simulink model "Lab2_simulink_plc"  (R2023b)
settings: Solver=FixedStepAuto  StartTime=0.0  StopTime=inf  RelTol=1e-3
InitFcn:
  | d13_init.PRE = 50;
undefined symbols (not defined in the file or in its folder): BOOL, DINT, OTL, slplc
libraries: arduinolib x9
(part 1 of 31)
settings Model: PLCLadderLogicDebug=off  PLCLadderLogicAnimation=on  PLCLadderLogicDataShow=off  ExtModeTrigDuration=2  Creator=pontus  LastModifiedBy=pontus
settings SolverCC: AllowMultiTaskInputOutput=on  ConcurrentTasks=on  AutoInsertRateTranBlk=on
settings OptimizationCC: EnhancedBackFolding=on  PassReuseOutputArgsAs=Individual arguments  ZeroExternalMemoryAtStartup=off  ZeroInternalMemoryAtStartup=off  MaxStackSize=64  OptimizeBlockOrder=speed  DifferentSizesBufferReuse=on  OptimizationCustomize=off  EfficientTunableParamExpr=on
settings DebuggingCC: ParameterTunabilityLossMsg=error  BusObjectLabelMismatch=error
settings HardwareCC: ProdBitPerInt=16  ProdBitPerPointer=16  ProdBitPerSizeT=16  ProdBitPerPtrDiffT=16  ProdLargestAtomicFloat=None  ProdWordSize=8  ProdHWDeviceType=Atmel->AVR  UseEmbeddedCoderFeatures=off  UseSimulinkCoderFeatures=off
settings SFSimCC: MATLABDynamicMemAlloc=off
settings RTWCC: Description=Embedded Coder  SystemTargetFile=ert.tlc  HardwareBoard=Arduino Uno  TemplateMakefile=ert_default_tmf  Toolchain=Arduino AVR
settings CodeAppCC: ForceParamTrailComments=on  IgnoreCustomStorageClasses=off  OperatorAnnotations=on  SimulinkDataObjDesc=on  SFDataObjDesc=on  InsertBlockDesc=on
settings ERTTargetCC: SupportContinuousTime=on  SupportNonInlinedSFcns=on  SupportVariableSizeSignals=on  ExtModeTransport=3  ExtModeStaticAlloc=on  ExtModeAutomaticAllocSize=off  ExtModeStaticAllocSize=250  ExtModeMexFile=ext_xcp  ExtModeIntrfLevel=Level2 - Open
settings ConfigComp: PLC_TargetIDE=studio5000  PLC_MultiTBSigbuilderTimeRange=off  PLC_FoldFBCallOutputVar=off  PLC_RemoveUnusedSSMethodCallArguments=off  PLC_UseCallOutputArgSyntax=off  PLC_EnableUnsignedAndDoubleTypes=off  PLC_EnableUDTSplitting=off  PLC_MaxFieldsInUDT=500  PLC_InlineEnumCastFunction=off

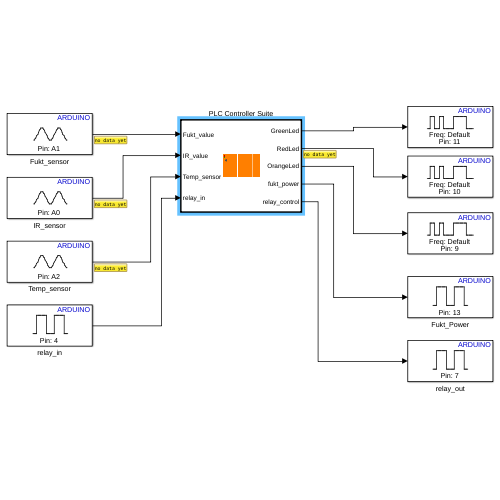
[thumbnail: Simulink's stored preview of the top-level diagram]
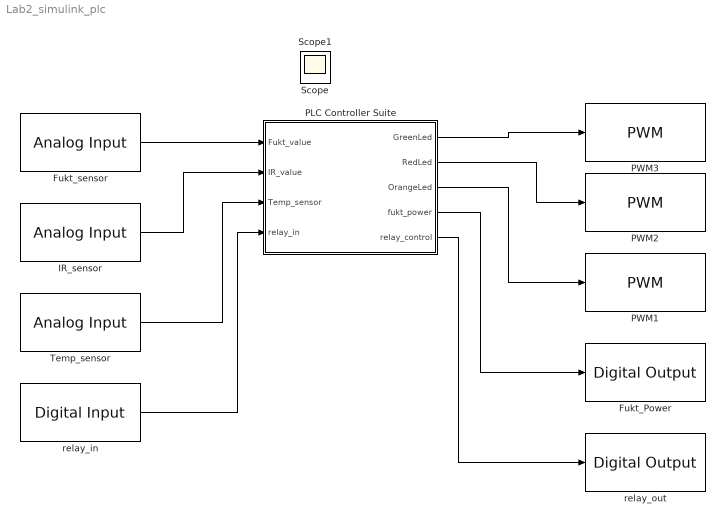
== system Lab2_simulink_plc ==
  Fukt_Power : arduinolib/Digital Output  pinNumber=13  Direction=output  blockPlatform=arduino
  Fukt_sensor : arduinolib/Analog Input  pinNumber=1  ReadResultsOnly=off  ConversionTriggerSource=Software  ExternalTriggerType=uint32( 0 )  OutputDataType=uint16  EnableConversionCompleteNotify=off  EnableOuputStatus=off  SampleTime=-1  blockPlatform=arduino
  IR_sensor : arduinolib/Analog Input  pinNumber=0  ReadResultsOnly=off  ConversionTriggerSource=Software  ExternalTriggerType=uint32( 0 )  OutputDataType=uint16  EnableConversionCompleteNotify=off  EnableOuputStatus=off  SampleTime=-1  blockPlatform=arduino
  PLC Controller Suite : Subsystem  TreatAsAtomicUnit=on  mask{PLCBlockType=PLCController, PLCPOUType=PLC Controller}
      mask init:
      | slplc.callbacks.initController(gcb);
      settings: OpenFcn=slplc.callbacks.openPOUBlock(gcb);
      mask Help: web([docroot '/plccoder/ref/plccontrollersuite' '.html']);
      mask DialogControl PLCBlockTitle Prompt: PLC Controller Block
  PWM1 : arduinolib/PWM  pinNumber=9  frequencyType=Default  desiredFrequency=490.2  achievableFrequency=490.1961
  PWM2 : arduinolib/PWM  pinNumber=10  frequencyType=Default  desiredFrequency=490.2  achievableFrequency=490.1961
  PWM3 : arduinolib/PWM  pinNumber=11  frequencyType=Default  desiredFrequency=490.2  achievableFrequency=490.2
  Temp_sensor : arduinolib/Analog Input  pinNumber=2  ReadResultsOnly=off  ConversionTriggerSource=Software  ExternalTriggerType=uint32( 0 )  OutputDataType=uint16  EnableConversionCompleteNotify=off  EnableOuputStatus=off  SampleTime=-1  blockPlatform=arduino
  relay_in : arduinolib/Digital Input  pinNumber=4  mode=Default  Direction=input  SampleTime=-1  blockPlatform=arduino
  relay_out : arduinolib/Digital Output  pinNumber=7  Direction=output  blockPlatform=arduino
  Scope : Scope
  Scope1 : Scope
  wires:
  PLC Controller Suite/1(GreenLed) -> PWM3/1
  PLC Controller Suite/2(RedLed) -> PWM2/1
  Fukt_sensor/1 -> PLC Controller Suite/1(Fukt_value)
  IR_sensor/1 -> PLC Controller Suite/2(IR_value)
  Temp_sensor/1 -> PLC Controller Suite/3(Temp_sensor)
  PLC Controller Suite/3(OrangeLed) -> PWM1/1
  PLC Controller Suite/4(fukt_power) -> Fukt_Power/1
  relay_in/1 -> PLC Controller Suite/4(relay_in)
  PLC Controller Suite/5(relay_control) -> relay_out/1

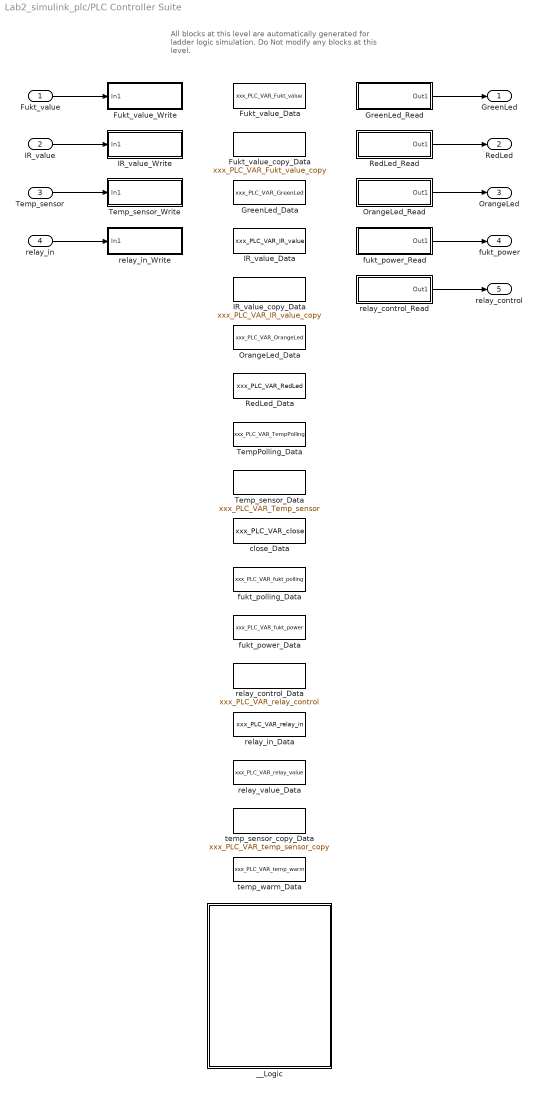
== system Lab2_simulink_plc/PLC Controller Suite ==
  note: All blocks at this level are automatically generated for ladder logic simulation.
      | Do Not modify any blocks at this level.
  Fukt_value : Inport  OutDataTypeStr=uint16
  IR_value : Inport  Port=2  OutDataTypeStr=uint16
  Temp_sensor : Inport  Port=3  OutDataTypeStr=uint16
  relay_in : Inport  Port=4  OutDataTypeStr=boolean
  Fukt_value_Data : DataStoreMemory  Tag=POUVariable  Priority=10  DataStoreName=xxx_PLC_VAR_Fukt_value  ShowAdditionalParam=off  OutDataTypeStr=uint16  Dimensions=1
  Fukt_value_Write : Subsystem  Priority=11  DisableCoverage=on  TreatAsAtomicUnit=on  mask{PLCOperandTag=Fukt_value, PLCOperandExpression=xxx_PLC_VAR_Fukt_value, PLCBlockType=VarWrite, PLCPOUType=VarWrite}
      mask prompts: PLCOperandTag "Operand Tag:"
      mask init:
      | slplc.callbacks.initInstruction(gcb);
      mask Help: web([docroot '/plccoder/ref/varwrite' '.html']);
      mask DialogControl PLCBlockTitle Prompt: Variable Write
      mask Display: disp(get_param(gcb, 'PLCOperandTag'));
  Fukt_value_copy_Data : DataStoreMemory  Tag=POUVariable  Priority=10  DataStoreName=xxx_PLC_VAR_Fukt_value_copy  ShowAdditionalParam=off  OutDataTypeStr=uint16  Dimensions=1
  GreenLed_Data : DataStoreMemory  Tag=POUVariable  Priority=10  DataStoreName=xxx_PLC_VAR_GreenLed  ShowAdditionalParam=off  OutDataTypeStr=uint8  Dimensions=1
  GreenLed_Read : Subsystem  Priority=13  DisableCoverage=on  TreatAsAtomicUnit=on  mask{PLCOperandTag=GreenLed, PLCOperandExpression=xxx_PLC_VAR_GreenLed, PLCBlockType=VarRead, PLCPOUType=VarRead}
      mask prompts: PLCOperandTag "Operand Tag"
      mask init:
      | block = gcb;
      | maskObj = Simulink.Mask.get(block);
      | maskObj.Display = sprintf('disp(''%s'');', PLCOperandTag);
      | slplc.callbacks.initInstruction(gcb);
      mask Help: web([docroot '/plccoder/ref/varread' '.html']);
      mask DialogControl PLCBlockTitle Prompt: Variable Read
      mask Display: disp('GreenLed');
  IR_value_Data : DataStoreMemory  Tag=POUVariable  Priority=10  DataStoreName=xxx_PLC_VAR_IR_value  ShowAdditionalParam=off  OutDataTypeStr=uint16  Dimensions=1
  IR_value_Write : Subsystem  Priority=11  DisableCoverage=on  TreatAsAtomicUnit=on  mask{PLCOperandTag=IR_value, PLCOperandExpression=xxx_PLC_VAR_IR_value, PLCBlockType=VarWrite, PLCPOUType=VarWrite}
      mask prompts: PLCOperandTag "Operand Tag:"
      mask init:
      | slplc.callbacks.initInstruction(gcb);
      mask Help: web([docroot '/plccoder/ref/varwrite' '.html']);
      mask DialogControl PLCBlockTitle Prompt: Variable Write
      mask Display: disp(get_param(gcb, 'PLCOperandTag'));
  IR_value_copy_Data : DataStoreMemory  Tag=POUVariable  Priority=10  DataStoreName=xxx_PLC_VAR_IR_value_copy  ShowAdditionalParam=off  OutDataTypeStr=uint16  Dimensions=1
  OrangeLed_Data : DataStoreMemory  Tag=POUVariable  Priority=10  DataStoreName=xxx_PLC_VAR_OrangeLed  ShowAdditionalParam=off  OutDataTypeStr=uint8  Dimensions=1
  OrangeLed_Read : Subsystem  Priority=13  DisableCoverage=on  TreatAsAtomicUnit=on  mask{PLCOperandTag=OrangeLed, PLCOperandExpression=xxx_PLC_VAR_OrangeLed, PLCBlockType=VarRead, PLCPOUType=VarRead}
      mask prompts: PLCOperandTag "Operand Tag"
      mask init:
      | block = gcb;
      | maskObj = Simulink.Mask.get(block);
      | maskObj.Display = sprintf('disp(''%s'');', PLCOperandTag);
      | slplc.callbacks.initInstruction(gcb);
      mask Help: web([docroot '/plccoder/ref/varread' '.html']);
      mask DialogControl PLCBlockTitle Prompt: Variable Read
      mask Display: disp('OrangeLed');
  RedLed_Data : DataStoreMemory  Tag=POUVariable  Priority=10  DataStoreName=xxx_PLC_VAR_RedLed  ShowAdditionalParam=off  OutDataTypeStr=uint8  Dimensions=1
  RedLed_Read : Subsystem  Priority=13  DisableCoverage=on  TreatAsAtomicUnit=on  mask{PLCOperandTag=RedLed, PLCOperandExpression=xxx_PLC_VAR_RedLed, PLCBlockType=VarRead, PLCPOUType=VarRead}
      mask prompts: PLCOperandTag "Operand Tag"
      mask init:
      | block = gcb;
      | maskObj = Simulink.Mask.get(block);
      | maskObj.Display = sprintf('disp(''%s'');', PLCOperandTag);
      | slplc.callbacks.initInstruction(gcb);
      mask Help: web([docroot '/plccoder/ref/varread' '.html']);
      mask DialogControl PLCBlockTitle Prompt: Variable Read
      mask Display: disp('RedLed');
  TempPolling_Data : DataStoreMemory  Tag=POUVariable  Priority=10  DataStoreName=xxx_PLC_VAR_TempPolling  ShowAdditionalParam=off  OutDataTypeStr=boolean  Dimensions=1
  Temp_sensor_Data : DataStoreMemory  Tag=POUVariable  Priority=10  DataStoreName=xxx_PLC_VAR_Temp_sensor  ShowAdditionalParam=off  OutDataTypeStr=uint16  Dimensions=1
  Temp_sensor_Write : Subsystem  Priority=11  DisableCoverage=on  TreatAsAtomicUnit=on  mask{PLCOperandTag=Temp_sensor, PLCOperandExpression=xxx_PLC_VAR_Temp_sensor, PLCBlockType=VarWrite, PLCPOUType=VarWrite}
      mask prompts: PLCOperandTag "Operand Tag:"
      mask init:
      | slplc.callbacks.initInstruction(gcb);
      mask Help: web([docroot '/plccoder/ref/varwrite' '.html']);
      mask DialogControl PLCBlockTitle Prompt: Variable Write
      mask Display: disp(get_param(gcb, 'PLCOperandTag'));
  __Logic : Subsystem  Priority=12  TreatAsAtomicUnit=on
  close_Data : DataStoreMemory  Tag=POUVariable  Priority=10  DataStoreName=xxx_PLC_VAR_close  ShowAdditionalParam=off  OutDataTypeStr=boolean  Dimensions=1
  fukt_polling_Data : DataStoreMemory  Tag=POUVariable  Priority=10  DataStoreName=xxx_PLC_VAR_fukt_polling  ShowAdditionalParam=off  OutDataTypeStr=boolean  Dimensions=1
  fukt_power_Data : DataStoreMemory  Tag=POUVariable  Priority=10  DataStoreName=xxx_PLC_VAR_fukt_power  ShowAdditionalParam=off  OutDataTypeStr=boolean  Dimensions=1
  fukt_power_Read : Subsystem  Priority=13  DisableCoverage=on  TreatAsAtomicUnit=on  mask{PLCOperandTag=fukt_power, PLCOperandExpression=xxx_PLC_VAR_fukt_power, PLCBlockType=VarRead, PLCPOUType=VarRead}
      mask prompts: PLCOperandTag "Operand Tag"
      mask init:
      | block = gcb;
      | maskObj = Simulink.Mask.get(block);
      | maskObj.Display = sprintf('disp(''%s'');', PLCOperandTag);
      | slplc.callbacks.initInstruction(gcb);
      mask Help: web([docroot '/plccoder/ref/varread' '.html']);
      mask DialogControl PLCBlockTitle Prompt: Variable Read
      mask Display: disp('fukt_power');
  relay_control_Data : DataStoreMemory  Tag=POUVariable  Priority=10  DataStoreName=xxx_PLC_VAR_relay_control  InitialValue=1  ShowAdditionalParam=off  OutDataTypeStr=boolean  Dimensions=1
  relay_control_Read : Subsystem  Priority=13  DisableCoverage=on  TreatAsAtomicUnit=on  mask{PLCOperandTag=relay_control, PLCOperandExpression=xxx_PLC_VAR_relay_control, PLCBlockType=VarRead, PLCPOUType=VarRead}
      mask prompts: PLCOperandTag "Operand Tag"
      mask init:
      | block = gcb;
      | maskObj = Simulink.Mask.get(block);
      | maskObj.Display = sprintf('disp(''%s'');', PLCOperandTag);
      | slplc.callbacks.initInstruction(gcb);
      mask Help: web([docroot '/plccoder/ref/varread' '.html']);
      mask DialogControl PLCBlockTitle Prompt: Variable Read
      mask Display: disp('relay_control');
  relay_in_Data : DataStoreMemory  Tag=POUVariable  Priority=10  DataStoreName=xxx_PLC_VAR_relay_in  ShowAdditionalParam=off  OutDataTypeStr=boolean  Dimensions=1
  relay_in_Write : Subsystem  Priority=11  DisableCoverage=on  TreatAsAtomicUnit=on  mask{PLCOperandTag=relay_in, PLCOperandExpression=xxx_PLC_VAR_relay_in, PLCBlockType=VarWrite, PLCPOUType=VarWrite}
      mask prompts: PLCOperandTag "Operand Tag:"
      mask init:
      | slplc.callbacks.initInstruction(gcb);
      mask Help: web([docroot '/plccoder/ref/varwrite' '.html']);
      mask DialogControl PLCBlockTitle Prompt: Variable Write
      mask Display: disp(get_param(gcb, 'PLCOperandTag'));
  relay_value_Data : DataStoreMemory  Tag=POUVariable  Priority=10  DataStoreName=xxx_PLC_VAR_relay_value  ShowAdditionalParam=off  OutDataTypeStr=boolean  Dimensions=1
  temp_sensor_copy_Data : DataStoreMemory  Tag=POUVariable  Priority=10  DataStoreName=xxx_PLC_VAR_temp_sensor_copy  ShowAdditionalParam=off  OutDataTypeStr=uint16  Dimensions=1
  temp_warm_Data : DataStoreMemory  Tag=POUVariable  Priority=10  DataStoreName=xxx_PLC_VAR_temp_warm  ShowAdditionalParam=off  OutDataTypeStr=boolean  Dimensions=1
  GreenLed : Outport
  RedLed : Outport  Port=2
  OrangeLed : Outport  Port=3
  fukt_power : Outport  Port=4
  relay_control : Outport  Port=5
  wires:
  GreenLed_Read/1(Out1) -> GreenLed/1
  RedLed_Read/1(Out1) -> RedLed/1
  Fukt_value/1 -> Fukt_value_Write/1(In1)
  IR_value/1 -> IR_value_Write/1(In1)
  Temp_sensor/1 -> Temp_sensor_Write/1(In1)
  OrangeLed_Read/1(Out1) -> OrangeLed/1
  fukt_power_Read/1(Out1) -> fukt_power/1
  relay_in/1 -> relay_in_Write/1(In1)
  relay_control_Read/1(Out1) -> relay_control/1

== system Lab2_simulink_plc/PLC Controller Suite/Fukt_value_Write ==
  In1 : Inport
  __CoreVarWrite : Subsystem  Tag=CoreVarWriteBlock  DisableCoverage=on  TreatAsAtomicUnit=on  mask{PLCBlockType=CoreVarWrite}
      mask init:
      | slplc.callbacks.initCoreVariable(gcb, 'write');
      mask DialogControl PLCBlockTitle Prompt: Core Variable Write
  wires:
  In1/1 -> __CoreVarWrite/1(u)

== system Lab2_simulink_plc/PLC Controller Suite/GreenLed_Read ==
  __CoreVarRead : Subsystem  Tag=CoreVarReadBlock  DisableCoverage=on  TreatAsAtomicUnit=on  mask{PLCBlockType=CoreVarRead}
      mask init:
      | slplc.callbacks.initCoreVariable(gcb, 'read');
      mask DialogControl PLCBlockTitle Prompt: Core Variable Read
  Out1 : Outport  VectorParamsAs1DForOutWhenUnconnected=off
  wires:
  __CoreVarRead/1(y) -> Out1/1

== system Lab2_simulink_plc/PLC Controller Suite/IR_value_Write ==
  In1 : Inport
  __CoreVarWrite : Subsystem  Tag=CoreVarWriteBlock  DisableCoverage=on  TreatAsAtomicUnit=on  mask{PLCBlockType=CoreVarWrite}
      mask init:
      | slplc.callbacks.initCoreVariable(gcb, 'write');
      mask DialogControl PLCBlockTitle Prompt: Core Variable Write
  wires:
  In1/1 -> __CoreVarWrite/1(u)

== system Lab2_simulink_plc/PLC Controller Suite/OrangeLed_Read ==
  __CoreVarRead : Subsystem  Tag=CoreVarReadBlock  DisableCoverage=on  TreatAsAtomicUnit=on  mask{PLCBlockType=CoreVarRead}
      mask init:
      | slplc.callbacks.initCoreVariable(gcb, 'read');
      mask DialogControl PLCBlockTitle Prompt: Core Variable Read
  Out1 : Outport  VectorParamsAs1DForOutWhenUnconnected=off
  wires:
  __CoreVarRead/1(y) -> Out1/1

== system Lab2_simulink_plc/PLC Controller Suite/RedLed_Read ==
  __CoreVarRead : Subsystem  Tag=CoreVarReadBlock  DisableCoverage=on  TreatAsAtomicUnit=on  mask{PLCBlockType=CoreVarRead}
      mask init:
      | slplc.callbacks.initCoreVariable(gcb, 'read');
      mask DialogControl PLCBlockTitle Prompt: Core Variable Read
  Out1 : Outport  VectorParamsAs1DForOutWhenUnconnected=off
  wires:
  __CoreVarRead/1(y) -> Out1/1

== system Lab2_simulink_plc/PLC Controller Suite/Temp_sensor_Write ==
  In1 : Inport
  __CoreVarWrite : Subsystem  Tag=CoreVarWriteBlock  DisableCoverage=on  TreatAsAtomicUnit=on  mask{PLCBlockType=CoreVarWrite}
      mask init:
      | slplc.callbacks.initCoreVariable(gcb, 'write');
      mask DialogControl PLCBlockTitle Prompt: Core Variable Write
  wires:
  In1/1 -> __CoreVarWrite/1(u)

== system Lab2_simulink_plc/PLC Controller Suite/__Logic ==
  note: Relä är true (1) initialt
  Controller Tags : Subsystem  Tag=ControllerVariableSS  DisableCoverage=on  TreatAsAtomicUnit=on  mask{PLCVarFukt_valueName=Fukt_value, PLCVarFukt_valueAddress=<empty>, PLCVarFukt_valueMapping=Input Symbol, PLCVarFukt_valuePortIndex=1, PLCVarFukt_valueDataType=uint16, PLCVarFukt_valueSize=1, PLCVarFukt_valueInitialValue=0, PLCVarFukt_valueToDelete=off, PLCVarIR_valueName=IR_value, PLCVarIR_valueAddress=<empty>, PLCVarIR_valueMapping=Input Symbol, PLCVarIR_valuePortIndex=2, PLCVarIR_valueDataType=uint16, PLCVarIR_valueSize=1, PLCVarIR_valueInitialValue=0, PLCVarIR_valueToDelete=off, PLCVarTemp_sensorName=Temp_sensor, PLCVarTemp_sensorAddress=<empty>, PLCVarTemp_sensorMapping=Input Symbol, PLCVarTemp_sensorPortIndex=3, PLCVarTemp_sensorDataType=uint16, PLCVarTemp_sensorSize=1, PLCVarTemp_sensorInitialValue=0, PLCVarTemp_sensorToDelete=off, PLCVarrelay_inName=relay_in, PLCVarrelay_inAddress=<empty>, PLCVarrelay_inMapping=Input Symbol, PLCVarrelay_inPortIndex=4, PLCVarrelay_inDataType=boolean, PLCVarrelay_inSize=1, PLCVarrelay_inInitialValue=0, PLCVarrelay_inToDelete=off, PLCVarGreenLedName=GreenLed, PLCVarGreenLedAddress=<empty>, PLCVarGreenLedMapping=Output Symbol, PLCVarGreenLedPortIndex=1, PLCVarGreenLedDataType=uint8, PLCVarGreenLedSize=1, PLCVarGreenLedInitialValue=0, PLCVarGreenLedToDelete=off, PLCVarRedLedName=RedLed, PLCVarRedLedAddress=<empty>, PLCVarRedLedMapping=Output Symbol, PLCVarRedLedPortIndex=2, PLCVarRedLedDataType=uint8, PLCVarRedLedSize=1, PLCVarRedLedInitialValue=0, PLCVarRedLedToDelete=off, PLCVarOrangeLedName=OrangeLed, PLCVarOrangeLedAddress=<empty>, PLCVarOrangeLedMapping=Output Symbol, PLCVarOrangeLedPortIndex=3, PLCVarOrangeLedDataType=uint8, PLCVarOrangeLedSize=1, PLCVarOrangeLedInitialValue=0, PLCVarOrangeLedToDelete=off, PLCVarfukt_powerName=fukt_power, PLCVarfukt_powerAddress=<empty>, PLCVarfukt_powerMapping=Output Symbol, PLCVarfukt_powerPortIndex=4, PLCVarfukt_powerDataType=boolean, PLCVarfukt_powerSize=1, PLCVarfukt_powerInitialValue=0, PLCVarfukt_powerToDelete=off, PLCVarrelay_controlName=relay_control, PLCVarrelay_controlAddress=<empty>, PLCVarrelay_controlMapping=Output Symbol, PLCVarrelay_controlPortIndex=5, PLCVarrelay_controlDataType=boolean, PLCVarrelay_controlSize=1, PLCVarrelay_controlInitialValue=1, PLCVarrelay_controlToDelete=off, PLCVarFukt_value_copyName=Fukt_value_copy, PLCVarFukt_value_copyAddress=<empty>, PLCVarFukt_value_copyMapping=Global Variable, PLCVarFukt_value_copyPortIndex=17, PLCVarFukt_value_copyDataType=uint16, PLCVarFukt_value_copySize=1, PLCVarFukt_value_copyInitialValue=0, PLCVarFukt_value_copyToDelete=off, PLCVarIR_value_copyName=IR_value_copy, PLCVarIR_value_copyAddress=<empty>, PLCVarIR_value_copyMapping=Global Variable, PLCVarIR_value_copyPortIndex=17, PLCVarIR_value_copyDataType=uint16, PLCVarIR_value_copySize=1, PLCVarIR_value_copyInitialValue=0, PLCVarIR_value_copyToDelete=off, PLCVarTempPollingName=TempPolling, PLCVarTempPollingAddress=<empty>, PLCVarTempPollingMapping=Global Variable, PLCVarTempPollingPortIndex=17, PLCVarTempPollingDataType=boolean, PLCVarTempPollingSize=1, PLCVarTempPollingInitialValue=0, PLCVarTempPollingToDelete=off, PLCVarcloseName=close, PLCVarcloseAddress=<empty>, PLCVarcloseMapping=Global Variable, PLCVarclosePortIndex=17, PLCVarcloseDataType=boolean, PLCVarcloseSize=1, PLCVarcloseInitialValue=0, PLCVarcloseToDelete=off, PLCVarfukt_pollingName=fukt_polling, PLCVarfukt_pollingAddress=<empty>, PLCVarfukt_pollingMapping=Global Variable, PLCVarfukt_pollingPortIndex=17, PLCVarfukt_pollingDataType=boolean, PLCVarfukt_pollingSize=1, PLCVarfukt_pollingInitialValue=0, PLCVarfukt_pollingToDelete=off, PLCVarrelay_valueName=relay_value, PLCVarrelay_valueAddress=<empty>, PLCVarrelay_valueMapping=Global Variable, PLCVarrelay_valuePortIndex=17, PLCVarrelay_valueDataType=boolean, PLCVarrelay_valueSize=1, PLCVarrelay_valueInitialValue=0, PLCVarrelay_valueToDelete=off, PLCVartemp_sensor_copyName=temp_sensor_copy, PLCVartemp_sensor_copyAddress=<empty>, PLCVartemp_sensor_copyMapping=Global Variable, PLCVartemp_sensor_copyPortIndex=17, PLCVartemp_sensor_copyDataType=uint16, PLCVartemp_sensor_copySize=1, PLCVartemp_sensor_copyInitialValue=0, PLCVartemp_sensor_copyToDelete=off, PLCVartemp_warmName=temp_warm, PLCVartemp_warmAddress=<empty>, PLCVartemp_warmMapping=Global Variable, PLCVartemp_warmPortIndex=17, PLCVartemp_warmDataType=boolean, PLCVartemp_warmSize=1, PLCVartemp_warmInitialValue=0, PLCVartemp_warmToDelete=off}
      mask init:
      | slplc.callbacks.initControllerVarSS(gcb);
      settings: OpenFcn=slplc.callbacks.openControllerVariableSS(gcb);
      mask Help: web([docroot '/plccoder/ref/controllertags' '.html']);
      mask TypeOptions PLCVarFukt_valueMapping: Global Variable | Input Symbol | Output Symbol
      mask Callback PLCVarFukt_valueMapping: slplc.callbacks.updateControllerVarSS(gcb);
      mask TypeOptions PLCVarFukt_valuePortIndex: 1 | 2 | 3 | 4 | 5 | 6 | 7 | 8 | 9 | 10 | 11 | 12 | 13 | 14 | 15 | 16 | 17
      mask TypeOptions PLCVarIR_valueMapping: Global Variable | Input Symbol | Output Symbol
      mask Callback PLCVarIR_valueMapping: slplc.callbacks.updateControllerVarSS(gcb);
      mask TypeOptions PLCVarIR_valuePortIndex: 1 | 2 | 3 | 4 | 5 | 6 | 7 | 8 | 9 | 10 | 11 | 12 | 13 | 14 | 15 | 16 | 17
      mask TypeOptions PLCVarTemp_sensorMapping: Global Variable | Input Symbol | Output Symbol
      mask Callback PLCVarTemp_sensorMapping: slplc.callbacks.updateControllerVarSS(gcb);
      mask TypeOptions PLCVarTemp_sensorPortIndex: 1 | 2 | 3 | 4 | 5 | 6 | 7 | 8 | 9 | 10 | 11 | 12 | 13 | 14 | 15 | 16 | 17
      mask TypeOptions PLCVarrelay_inMapping: Global Variable | Input Symbol | Output Symbol
      mask Callback PLCVarrelay_inMapping: slplc.callbacks.updateControllerVarSS(gcb);
      mask TypeOptions PLCVarrelay_inPortIndex: 1 | 2 | 3 | 4 | 5 | 6 | 7 | 8 | 9 | 10 | 11 | 12 | 13 | 14 | 15 | 16 | 17
      mask TypeOptions PLCVarGreenLedMapping: Global Variable | Input Symbol | Output Symbol
      mask Callback PLCVarGreenLedMapping: slplc.callbacks.updateControllerVarSS(gcb);
      mask TypeOptions PLCVarGreenLedPortIndex: 1 | 2 | 3 | 4 | 5 | 6 | 7 | 8 | 9 | 10 | 11 | 12 | 13 | 14 | 15 | 16 | 17
      mask TypeOptions PLCVarRedLedMapping: Global Variable | Input Symbol | Output Symbol
      mask Callback PLCVarRedLedMapping: slplc.callbacks.updateControllerVarSS(gcb);
      mask TypeOptions PLCVarRedLedPortIndex: 1 | 2 | 3 | 4 | 5 | 6 | 7 | 8 | 9 | 10 | 11 | 12 | 13 | 14 | 15 | 16 | 17
      mask TypeOptions PLCVarOrangeLedMapping: Global Variable | Input Symbol | Output Symbol
      mask Callback PLCVarOrangeLedMapping: slplc.callbacks.updateControllerVarSS(gcb);
      mask TypeOptions PLCVarOrangeLedPortIndex: 1 | 2 | 3 | 4 | 5 | 6 | 7 | 8 | 9 | 10 | 11 | 12 | 13 | 14 | 15 | 16 | 17
      mask TypeOptions PLCVarfukt_powerMapping: Global Variable | Input Symbol | Output Symbol
      mask Callback PLCVarfukt_powerMapping: slplc.callbacks.updateControllerVarSS(gcb);
      mask TypeOptions PLCVarfukt_powerPortIndex: 1 | 2 | 3 | 4 | 5 | 6 | 7 | 8 | 9 | 10 | 11 | 12 | 13 | 14 | 15 | 16 | 17
      mask TypeOptions PLCVarrelay_controlMapping: Global Variable | Input Symbol | Output Symbol
      mask Callback PLCVarrelay_controlMapping: slplc.callbacks.updateControllerVarSS(gcb);
      mask TypeOptions PLCVarrelay_controlPortIndex: 1 | 2 | 3 | 4 | 5 | 6 | 7 | 8 | 9 | 10 | 11 | 12 | 13 | 14 | 15 | 16 | 17
      mask TypeOptions PLCVarFukt_value_copyMapping: Global Variable | Input Symbol | Output Symbol
      mask Callback PLCVarFukt_value_copyMapping: slplc.callbacks.updateControllerVarSS(gcb);
      mask TypeOptions PLCVarFukt_value_copyPortIndex: 1 | 2 | 3 | 4 | 5 | 6 | 7 | 8 | 9 | 10 | 11 | 12 | 13 | 14 | 15 | 16 | 17
      mask TypeOptions PLCVarIR_value_copyMapping: Global Variable | Input Symbol | Output Symbol
      mask Callback PLCVarIR_value_copyMapping: slplc.callbacks.updateControllerVarSS(gcb);
      mask TypeOptions PLCVarIR_value_copyPortIndex: 1 | 2 | 3 | 4 | 5 | 6 | 7 | 8 | 9 | 10 | 11 | 12 | 13 | 14 | 15 | 16 | 17
      mask TypeOptions PLCVarTempPollingMapping: Global Variable | Input Symbol | Output Symbol
      mask Callback PLCVarTempPollingMapping: slplc.callbacks.updateControllerVarSS(gcb);
      mask TypeOptions PLCVarTempPollingPortIndex: 1 | 2 | 3 | 4 | 5 | 6 | 7 | 8 | 9 | 10 | 11 | 12 | 13 | 14 | 15 | 16 | 17
      mask TypeOptions PLCVarcloseMapping: Global Variable | Input Symbol | Output Symbol
      mask Callback PLCVarcloseMapping: slplc.callbacks.updateControllerVarSS(gcb);
      mask TypeOptions PLCVarclosePortIndex: 1 | 2 | 3 | 4 | 5 | 6 | 7 | 8 | 9 | 10 | 11 | 12 | 13 | 14 | 15 | 16 | 17
      mask TypeOptions PLCVarfukt_pollingMapping: Global Variable | Input Symbol | Output Symbol
      mask Callback PLCVarfukt_pollingMapping: slplc.callbacks.updateControllerVarSS(gcb);
      mask TypeOptions PLCVarfukt_pollingPortIndex: 1 | 2 | 3 | 4 | 5 | 6 | 7 | 8 | 9 | 10 | 11 | 12 | 13 | 14 | 15 | 16 | 17
      mask TypeOptions PLCVarrelay_valueMapping: Global Variable | Input Symbol | Output Symbol
      mask Callback PLCVarrelay_valueMapping: slplc.callbacks.updateControllerVarSS(gcb);
      mask TypeOptions PLCVarrelay_valuePortIndex: 1 | 2 | 3 | 4 | 5 | 6 | 7 | 8 | 9 | 10 | 11 | 12 | 13 | 14 | 15 | 16 | 17
      mask TypeOptions PLCVartemp_sensor_copyMapping: Global Variable | Input Symbol | Output Symbol
      mask Callback PLCVartemp_sensor_copyMapping: slplc.callbacks.updateControllerVarSS(gcb);
      mask TypeOptions PLCVartemp_sensor_copyPortIndex: 1 | 2 | 3 | 4 | 5 | 6 | 7 | 8 | 9 | 10 | 11 | 12 | 13 | 14 | 15 | 16 | 17
      mask TypeOptions PLCVartemp_warmMapping: Global Variable | Input Symbol | Output Symbol
      mask Callback PLCVartemp_warmMapping: slplc.callbacks.updateControllerVarSS(gcb);
      mask TypeOptions PLCVartemp_warmPortIndex: 1 | 2 | 3 | 4 | 5 | 6 | 7 | 8 | 9 | 10 | 11 | 12 | 13 | 14 | 15 | 16 | 17
      mask DialogControl PLCBlockTitle Prompt: Global Variable and Symbol Spreadsheet
      mask DialogControl PLCVarListHeaderName Prompt: Name
      mask DialogControl PLCVarListHeaderAddress Prompt: Address (At)
      mask DialogControl PLCVarListHeaderMapping Prompt: Mapping Type
      mask DialogControl PLCVarListHeaderPortIndex Prompt: Port
      mask DialogControl PLCVarListHeaderDataType Prompt: Data Type
      mask DialogControl PLCVarListHeaderSize Prompt: Size
      mask DialogControl PLCVarListHeaderInitialValue Prompt: Initial Value
      mask DialogControl PLCVarListHeaderToDelete Prompt: Delete
  Fukt logic : Subsystem  TreatAsAtomicUnit=on  mask{PLCBlockType=Task, PLCPOUType=Task, PLCTaskWatchDog=0}
      mask init:
      | slplc.callbacks.initTask(gcb);
      settings: OpenFcn=slplc.callbacks.openTaskBlock(gcb);
      mask Help: web([docroot '/plccoder/ref/task' '.html']);
      mask DialogControl PLCBlockTitle Prompt: Task
  Fukt sampling : Subsystem  Tag=fukt_task  Description=Task som samplar fukt sensorn  TreatAsAtomicUnit=on  mask{PLCBlockType=Task, PLCPOUType=Task, PLCTaskWatchDog=0}
      mask init:
      | slplc.callbacks.initTask(gcb);
      settings: OpenFcn=slplc.callbacks.openTaskBlock(gcb);
      mask Help: web([docroot '/plccoder/ref/task' '.html']);
      mask DialogControl PLCBlockTitle Prompt: Task
  IR logic : Subsystem  TreatAsAtomicUnit=on  mask{PLCBlockType=Task, PLCPOUType=Task, PLCTaskWatchDog=0}
      mask init:
      | slplc.callbacks.initTask(gcb);
      settings: OpenFcn=slplc.callbacks.openTaskBlock(gcb);
      mask Help: web([docroot '/plccoder/ref/task' '.html']);
      mask DialogControl PLCBlockTitle Prompt: Task
  IR sampling : Subsystem  TreatAsAtomicUnit=on  mask{PLCBlockType=Task, PLCPOUType=Task, PLCTaskWatchDog=0}
      settings: OpenFcn=slplc.callbacks.openTaskBlock(gcb);
      mask Help: web([docroot '/plccoder/ref/task' '.html']);
      mask DialogControl PLCBlockTitle Prompt: Task
  Temp logic : Subsystem  TreatAsAtomicUnit=on  mask{PLCBlockType=Task, PLCPOUType=Task, PLCTaskWatchDog=0}
      mask init:
      | slplc.callbacks.initTask(gcb);
      settings: OpenFcn=slplc.callbacks.openTaskBlock(gcb);
      mask Help: web([docroot '/plccoder/ref/task' '.html']);
      mask DialogControl PLCBlockTitle Prompt: Task
  Temp sampling : Subsystem  TreatAsAtomicUnit=on  mask{PLCBlockType=Task, PLCPOUType=Task, PLCTaskWatchDog=0}
      mask init:
      | slplc.callbacks.initTask(gcb);
      settings: OpenFcn=slplc.callbacks.openTaskBlock(gcb);
      mask Help: web([docroot '/plccoder/ref/task' '.html']);
      mask DialogControl PLCBlockTitle Prompt: Task
  Up to Parent POU : Subsystem  DisableCoverage=on  TreatAsAtomicUnit=on  mask{PLCBlockType=OpenParentPOU}
      settings: OpenFcn=slplc.callbacks.openParentPOUBlock(gcb);
      mask Help: web([docroot '/plccoder/ref/uptoparentpou' '.html']);
      mask DialogControl PLCBlockTitle Prompt: Add Single Rung
  relay : Subsystem  TreatAsAtomicUnit=on  mask{PLCBlockType=Task, PLCPOUType=Task, PLCTaskWatchDog=0}
      mask init:
      | slplc.callbacks.initTask(gcb);
      settings: OpenFcn=slplc.callbacks.openTaskBlock(gcb);
      mask Help: web([docroot '/plccoder/ref/task' '.html']);
      mask DialogControl PLCBlockTitle Prompt: Task

== system Lab2_simulink_plc/PLC Controller Suite/fukt_power_Read ==
  __CoreVarRead : Subsystem  Tag=CoreVarReadBlock  DisableCoverage=on  TreatAsAtomicUnit=on  mask{PLCBlockType=CoreVarRead}
      mask init:
      | slplc.callbacks.initCoreVariable(gcb, 'read');
      mask DialogControl PLCBlockTitle Prompt: Core Variable Read
  Out1 : Outport  VectorParamsAs1DForOutWhenUnconnected=off
  wires:
  __CoreVarRead/1(y) -> Out1/1

== system Lab2_simulink_plc/PLC Controller Suite/relay_control_Read ==
  __CoreVarRead : Subsystem  Tag=CoreVarReadBlock  DisableCoverage=on  TreatAsAtomicUnit=on  mask{PLCBlockType=CoreVarRead}
      mask init:
      | slplc.callbacks.initCoreVariable(gcb, 'read');
      mask DialogControl PLCBlockTitle Prompt: Core Variable Read
  Out1 : Outport  VectorParamsAs1DForOutWhenUnconnected=off
  wires:
  __CoreVarRead/1(y) -> Out1/1

== system Lab2_simulink_plc/PLC Controller Suite/relay_in_Write ==
  In1 : Inport
  __CoreVarWrite : Subsystem  Tag=CoreVarWriteBlock  DisableCoverage=on  TreatAsAtomicUnit=on  mask{PLCBlockType=CoreVarWrite}
      mask init:
      | slplc.callbacks.initCoreVariable(gcb, 'write');
      mask DialogControl PLCBlockTitle Prompt: Core Variable Write
  wires:
  In1/1 -> __CoreVarWrite/1(u)

== system Lab2_simulink_plc/PLC Controller Suite/Fukt_value_Write/__CoreVarWrite ==
  u : Inport
  DataWrite : DataStoreWrite  DataStoreName=xxx_PLC_VAR_Fukt_value  IndexOptions=Index vector (dialog)  Indices=1  OutputSizes=1
  wires:
  u/1 -> DataWrite/1

== system Lab2_simulink_plc/PLC Controller Suite/GreenLed_Read/__CoreVarRead ==
  DataRead : DataStoreRead  DataStoreName=xxx_PLC_VAR_GreenLed  IndexOptions=Index vector (dialog)  Indices=1  OutputSizes=1
  y : Outport  VectorParamsAs1DForOutWhenUnconnected=off
  wires:
  DataRead/1 -> y/1

== system Lab2_simulink_plc/PLC Controller Suite/IR_value_Write/__CoreVarWrite ==
  u : Inport
  DataWrite : DataStoreWrite  DataStoreName=xxx_PLC_VAR_IR_value  IndexOptions=Index vector (dialog)  Indices=1  OutputSizes=1
  wires:
  u/1 -> DataWrite/1

== system Lab2_simulink_plc/PLC Controller Suite/OrangeLed_Read/__CoreVarRead ==
  DataRead : DataStoreRead  DataStoreName=xxx_PLC_VAR_OrangeLed  IndexOptions=Index vector (dialog)  Indices=1  OutputSizes=1
  y : Outport  VectorParamsAs1DForOutWhenUnconnected=off
  wires:
  DataRead/1 -> y/1

== system Lab2_simulink_plc/PLC Controller Suite/RedLed_Read/__CoreVarRead ==
  DataRead : DataStoreRead  DataStoreName=xxx_PLC_VAR_RedLed  IndexOptions=Index vector (dialog)  Indices=1  OutputSizes=1
  y : Outport  VectorParamsAs1DForOutWhenUnconnected=off
  wires:
  DataRead/1 -> y/1

== system Lab2_simulink_plc/PLC Controller Suite/Temp_sensor_Write/__CoreVarWrite ==
  u : Inport
  DataWrite : DataStoreWrite  DataStoreName=xxx_PLC_VAR_Temp_sensor  IndexOptions=Index vector (dialog)  Indices=1  OutputSizes=1
  wires:
  u/1 -> DataWrite/1

== system Lab2_simulink_plc/PLC Controller Suite/__Logic/Controller Tags ==

== system Lab2_simulink_plc/PLC Controller Suite/__Logic/Fukt logic ==
  Program : Subsystem  TreatAsAtomicUnit=on  mask{PLCPOUType=Program, PLCPOULanguage=LD, PLCBlockType=LDProgram}
      mask prompts: PLCPOUType "POU Type:"
      mask init:
      | slplc.callbacks.initProgram(gcb);
      settings: OpenFcn=slplc.callbacks.openPOUBlock(gcb);
      mask Help: web([docroot '/plccoder/ref/program' '.html']);
      mask DialogControl PLCBlockTitle Prompt: Program
      mask DialogControl PLCEditPOU Prompt: Edit POU
      mask DialogControl PLCEditInterface Prompt: Edit Variables
  Up to Parent POU : Subsystem  DisableCoverage=on  TreatAsAtomicUnit=on  mask{PLCBlockType=OpenParentPOU}
      settings: OpenFcn=slplc.callbacks.openParentPOUBlock(gcb);
      mask Help: web([docroot '/plccoder/ref/uptoparentpou' '.html']);
      mask DialogControl PLCBlockTitle Prompt: Add Single Rung

== system Lab2_simulink_plc/PLC Controller Suite/__Logic/Fukt sampling ==
  Fuktmätare sampling : Subsystem  TreatAsAtomicUnit=on  mask{PLCPOUType=Program, PLCPOULanguage=LD, PLCBlockType=LDProgram}
      mask prompts: PLCPOUType "POU Type:"
      mask init:
      | slplc.callbacks.initProgram(gcb);
      settings: OpenFcn=slplc.callbacks.openPOUBlock(gcb);
      mask Help: web([docroot '/plccoder/ref/program' '.html']);
      mask DialogControl PLCBlockTitle Prompt: Program
      mask DialogControl PLCEditPOU Prompt: Edit POU
      mask DialogControl PLCEditInterface Prompt: Edit Variables
  Up to Parent POU : Subsystem  DisableCoverage=on  TreatAsAtomicUnit=on  mask{PLCBlockType=OpenParentPOU}
      settings: OpenFcn=slplc.callbacks.openParentPOUBlock(gcb);
      mask Help: web([docroot '/plccoder/ref/uptoparentpou' '.html']);
      mask DialogControl PLCBlockTitle Prompt: Add Single Rung

== system Lab2_simulink_plc/PLC Controller Suite/__Logic/IR logic ==
  IR sensor logic : Subsystem  TreatAsAtomicUnit=on  mask{PLCPOUType=Program, PLCPOULanguage=LD, PLCBlockType=LDProgram}
      mask prompts: PLCPOUType "POU Type:"
      mask init:
      | slplc.callbacks.initProgram(gcb);
      settings: OpenFcn=slplc.callbacks.openPOUBlock(gcb);
      mask Help: web([docroot '/plccoder/ref/program' '.html']);
      mask DialogControl PLCBlockTitle Prompt: Program
      mask DialogControl PLCEditPOU Prompt: Edit POU
      mask DialogControl PLCEditInterface Prompt: Edit Variables
  Up to Parent POU : Subsystem  DisableCoverage=on  TreatAsAtomicUnit=on  mask{PLCBlockType=OpenParentPOU}
      settings: OpenFcn=slplc.callbacks.openParentPOUBlock(gcb);
      mask Help: web([docroot '/plccoder/ref/uptoparentpou' '.html']);
      mask DialogControl PLCBlockTitle Prompt: Add Single Rung

== system Lab2_simulink_plc/PLC Controller Suite/__Logic/IR sampling ==
  IR sampling : Subsystem  TreatAsAtomicUnit=on  mask{PLCPOUType=Program, PLCPOULanguage=LD, PLCBlockType=LDProgram}
      mask prompts: PLCPOUType "POU Type:"
      mask init:
      | slplc.callbacks.initProgram(gcb);
      settings: OpenFcn=slplc.callbacks.openPOUBlock(gcb);
      mask Help: web([docroot '/plccoder/ref/ldprogram' '.html']);
      mask DialogControl PLCBlockTitle Prompt: Program
      mask DialogControl PLCEditPOU Prompt: Edit POU
      mask DialogControl PLCEditInterface Prompt: Edit Variables
  Up to Parent POU : Subsystem  DisableCoverage=on  TreatAsAtomicUnit=on  mask{PLCBlockType=OpenParentPOU}
      settings: OpenFcn=slplc.callbacks.openParentPOUBlock(gcb);
      mask Help: web([docroot '/plccoder/ref/uptoparentpou' '.html']);
      mask DialogControl PLCBlockTitle Prompt: Add Single Rung

== system Lab2_simulink_plc/PLC Controller Suite/__Logic/Temp logic ==
  Termperature sensor logic : Subsystem  TreatAsAtomicUnit=on  mask{PLCPOUType=Program, PLCPOULanguage=LD, PLCBlockType=LDProgram}
      mask prompts: PLCPOUType "POU Type:"
      mask init:
      | slplc.callbacks.initProgram(gcb);
      settings: OpenFcn=slplc.callbacks.openPOUBlock(gcb);
      mask Help: web([docroot '/plccoder/ref/program' '.html']);
      mask DialogControl PLCBlockTitle Prompt: Program
      mask DialogControl PLCEditPOU Prompt: Edit POU
      mask DialogControl PLCEditInterface Prompt: Edit Variables
  Up to Parent POU : Subsystem  DisableCoverage=on  TreatAsAtomicUnit=on  mask{PLCBlockType=OpenParentPOU}
      settings: OpenFcn=slplc.callbacks.openParentPOUBlock(gcb);
      mask Help: web([docroot '/plccoder/ref/uptoparentpou' '.html']);
      mask DialogControl PLCBlockTitle Prompt: Add Single Rung

== system Lab2_simulink_plc/PLC Controller Suite/__Logic/Temp sampling ==
  Temperature sampling : Subsystem  TreatAsAtomicUnit=on  mask{PLCPOUType=Program, PLCPOULanguage=LD, PLCBlockType=LDProgram}
      mask prompts: PLCPOUType "POU Type:"
      mask init:
      | slplc.callbacks.initProgram(gcb);
      settings: OpenFcn=slplc.callbacks.openPOUBlock(gcb);
      mask Help: web([docroot '/plccoder/ref/program' '.html']);
      mask DialogControl PLCBlockTitle Prompt: Program
      mask DialogControl PLCEditPOU Prompt: Edit POU
      mask DialogControl PLCEditInterface Prompt: Edit Variables
  Up to Parent POU : Subsystem  DisableCoverage=on  TreatAsAtomicUnit=on  mask{PLCBlockType=OpenParentPOU}
      settings: OpenFcn=slplc.callbacks.openParentPOUBlock(gcb);
      mask Help: web([docroot '/plccoder/ref/uptoparentpou' '.html']);
      mask DialogControl PLCBlockTitle Prompt: Add Single Rung

== system Lab2_simulink_plc/PLC Controller Suite/__Logic/Up to Parent POU ==

== system Lab2_simulink_plc/PLC Controller Suite/__Logic/relay ==
  Up to Parent POU : Subsystem  DisableCoverage=on  TreatAsAtomicUnit=on  mask{PLCBlockType=OpenParentPOU}
      settings: OpenFcn=slplc.callbacks.openParentPOUBlock(gcb);
      mask Help: web([docroot '/plccoder/ref/uptoparentpou' '.html']);
      mask DialogControl PLCBlockTitle Prompt: Add Single Rung
  relay : Subsystem  TreatAsAtomicUnit=on  mask{PLCPOUType=Program, PLCPOULanguage=LD, PLCBlockType=LDProgram}
      mask prompts: PLCPOUType "POU Type:"
      mask init:
      | slplc.callbacks.initProgram(gcb);
      settings: OpenFcn=slplc.callbacks.openPOUBlock(gcb);
      mask Help: web([docroot '/plccoder/ref/program' '.html']);
      mask DialogControl PLCBlockTitle Prompt: Program
      mask DialogControl PLCEditPOU Prompt: Edit POU
      mask DialogControl PLCEditInterface Prompt: Edit Variables

== system Lab2_simulink_plc/PLC Controller Suite/fukt_power_Read/__CoreVarRead ==
  DataRead : DataStoreRead  DataStoreName=xxx_PLC_VAR_fukt_power  IndexOptions=Index vector (dialog)  Indices=1  OutputSizes=1
  y : Outport  VectorParamsAs1DForOutWhenUnconnected=off
  wires:
  DataRead/1 -> y/1

== system Lab2_simulink_plc/PLC Controller Suite/relay_control_Read/__CoreVarRead ==
  DataRead : DataStoreRead  DataStoreName=xxx_PLC_VAR_relay_control  IndexOptions=Index vector (dialog)  Indices=1  OutputSizes=1
  y : Outport  VectorParamsAs1DForOutWhenUnconnected=off
  wires:
  DataRead/1 -> y/1

== system Lab2_simulink_plc/PLC Controller Suite/relay_in_Write/__CoreVarWrite ==
  u : Inport
  DataWrite : DataStoreWrite  DataStoreName=xxx_PLC_VAR_relay_in  IndexOptions=Index vector (dialog)  Indices=1  OutputSizes=1
  wires:
  u/1 -> DataWrite/1

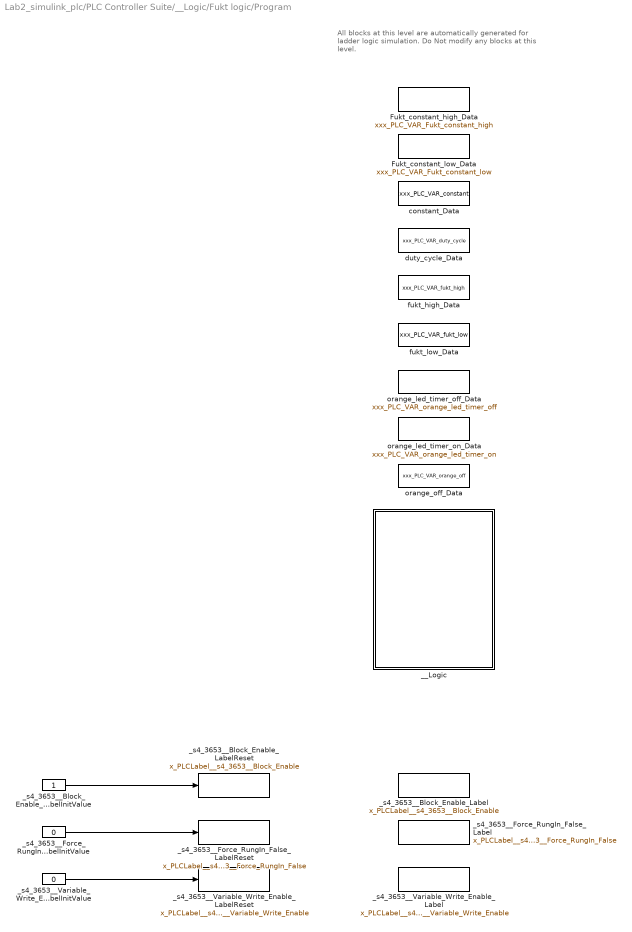
== system Lab2_simulink_plc/PLC Controller Suite/__Logic/Fukt logic/Program ==
  note: All blocks at this level are automatically generated for ladder logic simulation.
      | Do Not modify any blocks at this level.
  Fukt_constant_high_Data : DataStoreMemory  Tag=POUVariable  Priority=10  DataStoreName=xxx_PLC_VAR_Fukt_constant_high  InitialValue=900  ShowAdditionalParam=off  OutDataTypeStr=DINT  Dimensions=1
  Fukt_constant_low_Data : DataStoreMemory  Tag=POUVariable  Priority=10  DataStoreName=xxx_PLC_VAR_Fukt_constant_low  InitialValue=300  ShowAdditionalParam=off  OutDataTypeStr=DINT  Dimensions=1
  __Logic : Subsystem  Priority=12  TreatAsAtomicUnit=on
  _s4_3653__Block_Enable_Label : DataStoreMemory  Priority=10  DataStoreName=x_PLCLabel__s4_3653__Block_Enable  InitialValue=1  ShowAdditionalParam=off  OutDataTypeStr=BOOL  Dimensions=1
  _s4_3653__Block_Enable_LabelInitValue : Constant  OutDataTypeStr=BOOL
  _s4_3653__Block_Enable_LabelReset : DataStoreWrite  Priority=11  DataStoreName=x_PLCLabel__s4_3653__Block_Enable  IndexOptions=Index vector (dialog)  Indices=1  OutputSizes=1
  _s4_3653__Force_RungIn_False_Label : DataStoreMemory  Priority=10  DataStoreName=x_PLCLabel__s4_3653__Force_RungIn_False  ShowAdditionalParam=off  OutDataTypeStr=BOOL  Dimensions=1
  _s4_3653__Force_RungIn_False_LabelInitValue : Constant  Value=0  OutDataTypeStr=BOOL
  _s4_3653__Force_RungIn_False_LabelReset : DataStoreWrite  Priority=11  DataStoreName=x_PLCLabel__s4_3653__Force_RungIn_False  IndexOptions=Index vector (dialog)  Indices=1  OutputSizes=1
  _s4_3653__Variable_Write_Enable_Label : DataStoreMemory  Priority=10  DataStoreName=x_PLCLabel__s4_3653__Variable_Write_Enable  ShowAdditionalParam=off  OutDataTypeStr=BOOL  Dimensions=1
  _s4_3653__Variable_Write_Enable_LabelInitValue : Constant  Value=0  OutDataTypeStr=BOOL
  _s4_3653__Variable_Write_Enable_LabelReset : DataStoreWrite  Priority=11  DataStoreName=x_PLCLabel__s4_3653__Variable_Write_Enable  IndexOptions=Index vector (dialog)  Indices=1  OutputSizes=1
  constant_Data : DataStoreMemory  Tag=POUVariable  Priority=10  DataStoreName=xxx_PLC_VAR_constant  InitialValue=4  ShowAdditionalParam=off  OutDataTypeStr=uint16  Dimensions=1
  duty_cycle_Data : DataStoreMemory  Tag=POUVariable  Priority=10  DataStoreName=xxx_PLC_VAR_duty_cycle  ShowAdditionalParam=off  OutDataTypeStr=uint8  Dimensions=1
  fukt_high_Data : DataStoreMemory  Tag=POUVariable  Priority=10  DataStoreName=xxx_PLC_VAR_fukt_high  InitialValue=false  ShowAdditionalParam=off  OutDataTypeStr=BOOL  Dimensions=1
  fukt_low_Data : DataStoreMemory  Tag=POUVariable  Priority=10  DataStoreName=xxx_PLC_VAR_fukt_low  InitialValue=false  ShowAdditionalParam=off  OutDataTypeStr=BOOL  Dimensions=1
  orange_led_timer_off_Data : DataStoreMemory  Tag=POUVariable  Priority=10  DataStoreName=xxx_PLC_VAR_orange_led_timer_off  InitialValue=orange_period  ShowAdditionalParam=off  OutDataTypeStr=Bus: TIMER  Dimensions=1
  orange_led_timer_on_Data : DataStoreMemory  Tag=POUVariable  Priority=10  DataStoreName=xxx_PLC_VAR_orange_led_timer_on  InitialValue=orange_period  ShowAdditionalParam=off  OutDataTypeStr=Bus: TIMER  Dimensions=1
  orange_off_Data : DataStoreMemory  Tag=POUVariable  Priority=10  DataStoreName=xxx_PLC_VAR_orange_off  ShowAdditionalParam=off  OutDataTypeStr=uint8  Dimensions=1
  wires:
  _s4_3653__Block_Enable_LabelInitValue/1 -> _s4_3653__Block_Enable_LabelReset/1
  _s4_3653__Variable_Write_Enable_LabelInitValue/1 -> _s4_3653__Variable_Write_Enable_LabelReset/1
  _s4_3653__Force_RungIn_False_LabelInitValue/1 -> _s4_3653__Force_RungIn_False_LabelReset/1

== system Lab2_simulink_plc/PLC Controller Suite/__Logic/Fukt logic/Up to Parent POU ==

== system Lab2_simulink_plc/PLC Controller Suite/__Logic/Fukt sampling/Fuktmätare sampling ==
  note: All blocks at this level are automatically generated for ladder logic simulation.
      | Do Not modify any blocks at this level.
  Fukt_periodicity_Data : DataStoreMemory  Tag=POUVariable  Priority=10  DataStoreName=xxx_PLC_VAR_Fukt_periodicity  InitialValue=fukt_period  ShowAdditionalParam=off  OutDataTypeStr=Bus: TIMER  Dimensions=1
  __Logic : Subsystem  Priority=12  TreatAsAtomicUnit=on
  _s4_3653__Block_Enable_Label : DataStoreMemory  Priority=10  DataStoreName=x_PLCLabel__s4_3653__Block_Enable  InitialValue=1  ShowAdditionalParam=off  OutDataTypeStr=BOOL  Dimensions=1
  _s4_3653__Block_Enable_LabelInitValue : Constant  OutDataTypeStr=BOOL
  _s4_3653__Block_Enable_LabelReset : DataStoreWrite  Priority=11  DataStoreName=x_PLCLabel__s4_3653__Block_Enable  IndexOptions=Index vector (dialog)  Indices=1  OutputSizes=1
  _s4_3653__Force_RungIn_False_Label : DataStoreMemory  Priority=10  DataStoreName=x_PLCLabel__s4_3653__Force_RungIn_False  ShowAdditionalParam=off  OutDataTypeStr=BOOL  Dimensions=1
  _s4_3653__Force_RungIn_False_LabelInitValue : Constant  Value=0  OutDataTypeStr=BOOL
  _s4_3653__Force_RungIn_False_LabelReset : DataStoreWrite  Priority=11  DataStoreName=x_PLCLabel__s4_3653__Force_RungIn_False  IndexOptions=Index vector (dialog)  Indices=1  OutputSizes=1
  _s4_3653__Variable_Write_Enable_Label : DataStoreMemory  Priority=10  DataStoreName=x_PLCLabel__s4_3653__Variable_Write_Enable  ShowAdditionalParam=off  OutDataTypeStr=BOOL  Dimensions=1
  _s4_3653__Variable_Write_Enable_LabelInitValue : Constant  Value=0  OutDataTypeStr=BOOL
  _s4_3653__Variable_Write_Enable_LabelReset : DataStoreWrite  Priority=11  DataStoreName=x_PLCLabel__s4_3653__Variable_Write_Enable  IndexOptions=Index vector (dialog)  Indices=1  OutputSizes=1
  d13_wait_Data : DataStoreMemory  Tag=POUVariable  Priority=10  DataStoreName=xxx_PLC_VAR_d13_wait  InitialValue=d13_period  ShowAdditionalParam=off  OutDataTypeStr=Bus: TIMER  Dimensions=1
  wires:
  _s4_3653__Block_Enable_LabelInitValue/1 -> _s4_3653__Block_Enable_LabelReset/1
  _s4_3653__Variable_Write_Enable_LabelInitValue/1 -> _s4_3653__Variable_Write_Enable_LabelReset/1
  _s4_3653__Force_RungIn_False_LabelInitValue/1 -> _s4_3653__Force_RungIn_False_LabelReset/1

== system Lab2_simulink_plc/PLC Controller Suite/__Logic/Fukt sampling/Up to Parent POU ==
